simulink model "NREL_20MW_4TT"  (R2016b)
settings: Solver=VariableStepAuto  StartTime=0.0  StopTime=Tsim  FixedStep=Ts  RelTol=1e-3
values from neighbouring files (symbols this model uses):
  k1 = kgain(1,k)    [SimulationMain.m]
  k2 = kgain(2,k)    [SimulationMain.m]
  k3 = kgain(3,k)    [SimulationMain.m]
  k4 = kgain(4,k)    [SimulationMain.m]
  La02 = par.La02    [SimulationMain.m]
  La04 = par.La04    [SimulationMain.m]
  La12 = par.La12    [SimulationMain.m]
  La14 = par.La14    [SimulationMain.m]
  Lhub = par.Lhub    [SimulationMain.m]
  LNaz = par.LNaz    [SimulationMain.m]
  Lt1 = par.Lt1    [SimulationMain.m]
  Lt2 = par.Lt2    [SimulationMain.m]
  MRTCTs = 0.0125    [SimulationMain.m]
  NDOF = 10    [SimulationMain.m]
  Px_PowerMax = 6e6    (= 6000000)    [SimulationMain.m]
  ttilt = par.ttilt    [SimulationMain.m]
undefined symbols (not defined in the file or in its folder): A_4TT, B_4TT, C_4TT, D_4TT, DiscIntNeverNeededParam, Ks, Ms
libraries: libturbines x4
settings Model: Creator=kihso  LastModifiedBy=kihso

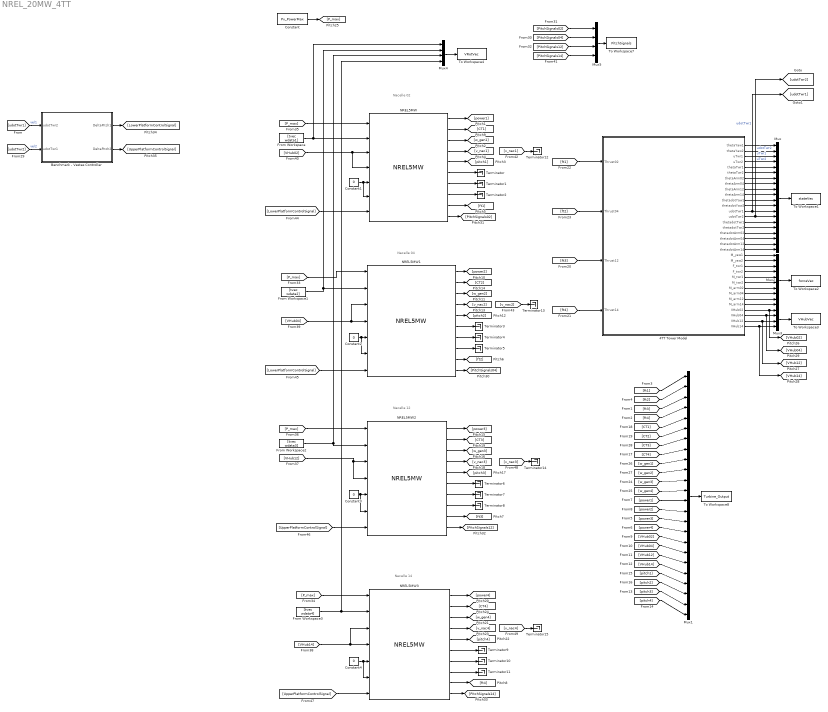
== system NREL_20MW_4TT ==
  note: Nacelle 02
  note: Nacelle 04
  note: Nacelle 12
  note: Nacelle 14
  4TT Tower Model : Subsystem
      mask Display: image(imread('Icon_4TT.JPG'))
  Benchmark - Vestas Controller : Subsystem
      mask Display: image('Vestas.png');
  Constant : Constant  Value=Px_PowerMax
  Constant{1..4} (4 alike) : Constant  Value=0
  From Workspace : FromWorkspace  VariableName=[tvec wdata1]  SampleTime=0
  From Workspace1 : FromWorkspace  VariableName=[tvec wdata2]  SampleTime=0
  From Workspace2 : FromWorkspace  VariableName=[tvec wdata3]  SampleTime=0
  From Workspace3 : FromWorkspace  VariableName=[tvec wdata4]  SampleTime=0
  Mux : Mux  Inputs=20
  Mux1 : Mux  Inputs=24
  Mux2 : Mux  Inputs=10
  Mux3 : Mux
  Mux4 : Mux
  Mux5 : Mux
  NREL5MW{,1..3} (4 alike) : libturbines/NREL5MW  w_gen_ini=50  beta_ini=0  auto=off  torq_ini=0
  Terminator{,1..15} (16 alike) : Terminator
  To Workspace1 : ToWorkspace  VariableName=stateVec  SaveFormat=Timeseries  SampleTime=-1
  To Workspace2 : ToWorkspace  VariableName=forceVec  SaveFormat=Timeseries  SampleTime=-1
  To Workspace3 : ToWorkspace  VariableName=VHubVec  SaveFormat=Timeseries  SampleTime=-1
  To Workspace4 : ToWorkspace  VariableName=VRotVec  SaveFormat=Timeseries  SampleTime=-1
  To Workspace7 : ToWorkspace  VariableName=PitchSignals  SaveFormat=Timeseries  SampleTime=-1
  To Workspace8 : ToWorkspace  VariableName=Turbine_Output  SaveFormat=Timeseries  SampleTime=-1
  wires:
  4TT Tower Model/1(thetaYaw1) -> Mux/1
  4TT Tower Model/2(thetaYaw2) -> Mux/2
  4TT Tower Model/3(uTwr1) -> Mux/3  "uTwr1"
  4TT Tower Model/4(uTwr2) -> Mux/4  "uTwr2"
  4TT Tower Model/5(thetaTwr1) -> Mux/5
  4TT Tower Model/6(thetaTwr2) -> Mux/6
  4TT Tower Model/7(thetaArm02) -> Mux/7
  4TT Tower Model/8(thetaArm04) -> Mux/8
  4TT Tower Model/9(thetaArm12) -> Mux/9
  4TT Tower Model/10(thetaArm14) -> Mux/10
  4TT Tower Model/11(thetadotYaw1) -> Mux/11
  4TT Tower Model/12(thetadotYaw2) -> Mux/12
  4TT Tower Model/13(udotTwr1) -> Mux/13, [udotTwr1]  "udotTwr1"
  4TT Tower Model/14(udotTwr2) -> Mux/14, [udotTwr2]  "udotTwr2"
  4TT Tower Model/15(thetadotTwr1) -> Mux/15
  4TT Tower Model/16(thetadotTwr2) -> Mux/16
  4TT Tower Model/17(thetadotArm02) -> Mux/17
  4TT Tower Model/18(thetadotArm04) -> Mux/18
  4TT Tower Model/19(thetadotArm12) -> Mux/19
  Mux1/1 -> To Workspace8/1
  NREL5MW/1 -> [power1]
  NREL5MW/2 -> [CT1]
  NREL5MW/3 -> [w_gen1]
  NREL5MW1/6 -> Terminator3/1
  NREL5MW1/7 -> Terminator4/1
  NREL5MW1/5 -> [pitch2]
  NREL5MW1/1 -> [power2]
  NREL5MW1/3 -> [w_gen2]
  NREL5MW1/2 -> [CT2]
  NREL5MW1/4 -> [v_nac2]
  NREL5MW2/6 -> Terminator6/1
  NREL5MW2/7 -> Terminator7/1
  NREL5MW2/2 -> [CT3]
  NREL5MW2/4 -> [v_nac3]
  NREL5MW2/1 -> [power3]
  NREL5MW2/3 -> [w_gen3]
  NREL5MW2/5 -> [pitch3]
  NREL5MW3/6 -> Terminator9/1
  NREL5MW3/7 -> Terminator10/1
  NREL5MW3/1 -> [power4]
  NREL5MW3/2 -> [CT4]
  NREL5MW3/4 -> [v_nac4]
  NREL5MW3/5 -> [pitch4]
  NREL5MW3/3 -> [w_gen4]
  Constant/1 -> [P_max]
  [P_max] -> NREL5MW/1
  [P_max] -> NREL5MW1/1
  [P_max] -> NREL5MW3/1
  From Workspace3/1 -> NREL5MW3/2, Mux4/4
  From Workspace2/1 -> NREL5MW2/2, Mux4/3
  From Workspace1/1 -> Mux4/2, NREL5MW1/2
  From Workspace/1 -> NREL5MW/2, Mux4/1
  4TT Tower Model/23(F_twr1) -> Mux2/3
  4TT Tower Model/26(M_twr2) -> Mux2/6
  4TT Tower Model/30(M_arm14) -> Mux2/10
  4TT Tower Model/28(M_arm04) -> Mux2/8
  4TT Tower Model/21(M_yaw1) -> Mux2/1
  4TT Tower Model/24(F_twr2) -> Mux2/4
  4TT Tower Model/25(M_twr1) -> Mux2/5
  4TT Tower Model/22(M_yaw2) -> Mux2/2
  4TT Tower Model/29(M_arm12) -> Mux2/9
  4TT Tower Model/27(M_arm02) -> Mux2/7
  Mux2/1 -> To Workspace2/1
  4TT Tower Model/31(VHub02) -> [VHub02], Mux3/1
  4TT Tower Model/32(VHub04) -> [VHub04], Mux3/2
  4TT Tower Model/33(VHub12) -> [VHub12], Mux3/3
  4TT Tower Model/34(VHub14) -> [VHub14], Mux3/4
  Mux3/1 -> To Workspace3/1
  [VHub04] -> NREL5MW1/4, NREL5MW1/3
  [VHub02] -> NREL5MW/4, NREL5MW/3
  NREL5MW1/8 -> Terminator5/1
  NREL5MW2/8 -> Terminator8/1
  NREL5MW3/8 -> Terminator11/1
  [ft1] -> 4TT Tower Model/1(Thrust02)
  [ft2] -> 4TT Tower Model/2(Thrust04)
  [ft3] -> 4TT Tower Model/3(Thrust12)
  [ft4] -> 4TT Tower Model/4(Thrust14)
  [VHub12] -> NREL5MW2/4, NREL5MW2/3
  [VHub14] -> NREL5MW3/4, NREL5MW3/3
  [w_gen1] -> Mux1/9
  [VHub04] -> Mux1/18
  [pitch2] -> Mux1/22
  [VHub12] -> Mux1/19
  [ft4] -> Mux1/4
  [ft1] -> Mux1/1
  [ft3] -> Mux1/3
  [CT4] -> Mux1/8
  [pitch3] -> Mux1/23
  [pitch4] -> Mux1/24
  [CT1] -> Mux1/5
  [CT2] -> Mux1/6
  [w_gen4] -> Mux1/12
  [pitch1] -> Mux1/21
  [w_gen2] -> Mux1/10
  [CT3] -> Mux1/7
  [VHub02] -> Mux1/17
  [w_gen3] -> Mux1/11
  [VHub14] -> Mux1/20
  [ft2] -> Mux1/2
  [power3] -> Mux1/15
  [power1] -> Mux1/13
  [power4] -> Mux1/16
  [power2] -> Mux1/14
  NREL5MW1/9 -> [ft2]
  NREL5MW2/9 -> [ft3]
  NREL5MW3/9 -> [ft4]
  Mux/1 -> To Workspace1/1
  Mux4/1 -> To Workspace4/1
  NREL5MW/4 -> [v_nac1]
  NREL5MW/5 -> [pitch1]
  NREL5MW/6 -> Terminator/1
  NREL5MW/7 -> Terminator1/1
  NREL5MW/8 -> Terminator2/1
  NREL5MW/9 -> [ft1]
  [P_max] -> NREL5MW2/1
  NREL5MW2/10 -> [PitchSignals12]
  NREL5MW3/10 -> [PitchSignals14]
  [PitchSignals02] -> Mux5/1
  [PitchSignals14] -> Mux5/4
  [PitchSignals04] -> Mux5/2
  [PitchSignals12] -> Mux5/3
  Mux5/1 -> To Workspace7/1
  4TT Tower Model/20(thetadotArm14) -> Mux/20
  [LowerPlatformControlSignal] -> NREL5MW/7
  [LowerPlatformControlSignal] -> NREL5MW1/7
  [UpperPlatformControlSignal] -> NREL5MW2/7
  [UpperPlatformControlSignal] -> NREL5MW3/7
  NREL5MW/10 -> [PitchSignals02]
  NREL5MW1/10 -> [PitchSignals04]
  Benchmark - Vestas Controller/1(DeltaPitch1) -> [LowerPlatformControlSignal]
  Benchmark - Vestas Controller/2(DeltaPitch2) -> [UpperPlatformControlSignal]
  [udotTwr1] -> Benchmark - Vestas Controller/1(udotTwr2)  "vel1"
  [udotTwr2] -> Benchmark - Vestas Controller/2(udotTwr1)  "vel2"
  Constant1/1 -> NREL5MW/6, NREL5MW/5
  Constant2/1 -> NREL5MW1/6, NREL5MW1/5
  Constant3/1 -> NREL5MW2/6, NREL5MW2/5
  Constant4/1 -> NREL5MW3/6, NREL5MW3/5
  [v_nac1] -> Terminator12/1
  [v_nac2] -> Terminator13/1
  [v_nac3] -> Terminator14/1
  [v_nac4] -> Terminator15/1

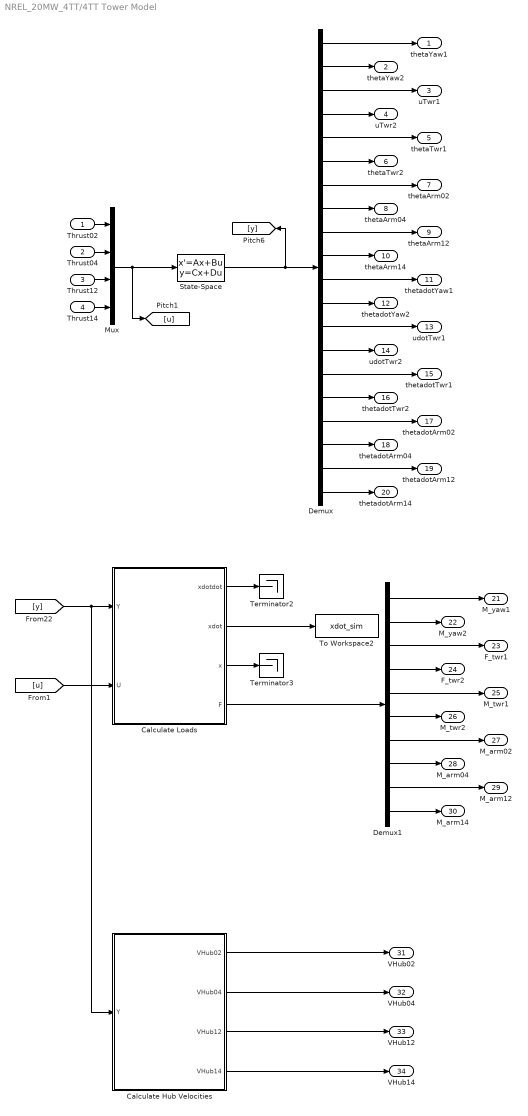
== system NREL_20MW_4TT/4TT Tower Model ==
  Thrust02 : Inport
  Thrust04 : Inport  Port=2
  Thrust12 : Inport  Port=3
  Thrust14 : Inport  Port=4
  Calculate Hub Velocities : MATLAB Function  TreatAsAtomicUnit=on
  Calculate Loads : MATLAB Function  TreatAsAtomicUnit=on
  Demux : Demux  Outputs=20
  Demux1 : Demux  Outputs=10
  Mux : Mux
  State-Space : StateSpace  A=A_4TT  B=B_4TT  C=C_4TT  D=D_4TT  X0=0
  Terminator2 : Terminator
  Terminator3 : Terminator
  To Workspace2 : ToWorkspace  VariableName=xdot_sim  SaveFormat=Timeseries  SampleTime=-1
  thetaYaw1 : Outport
  thetaYaw2 : Outport  Port=2
  uTwr1 : Outport  Port=3
  uTwr2 : Outport  Port=4
  thetaTwr1 : Outport  Port=5
  thetaTwr2 : Outport  Port=6
  thetaArm02 : Outport  Port=7
  thetaArm04 : Outport  Port=8
  thetaArm12 : Outport  Port=9
  thetaArm14 : Outport  Port=10
  thetadotYaw1 : Outport  Port=11
  thetadotYaw2 : Outport  Port=12
  udotTwr1 : Outport  Port=13
  udotTwr2 : Outport  Port=14
  thetadotTwr1 : Outport  Port=15
  thetadotTwr2 : Outport  Port=16
  thetadotArm02 : Outport  Port=17
  thetadotArm04 : Outport  Port=18
  thetadotArm12 : Outport  Port=19
  thetadotArm14 : Outport  Port=20
  M_yaw1 : Outport  Port=21
  M_yaw2 : Outport  Port=22
  F_twr1 : Outport  Port=23
  F_twr2 : Outport  Port=24
  M_twr1 : Outport  Port=25
  M_twr2 : Outport  Port=26
  M_arm02 : Outport  Port=27
  M_arm04 : Outport  Port=28
  M_arm12 : Outport  Port=29
  M_arm14 : Outport  Port=30
  VHub02 : Outport  Port=31
  VHub04 : Outport  Port=32
  VHub12 : Outport  Port=33
  VHub14 : Outport  Port=34
  wires:
  State-Space/1 -> [y], Demux/1
  Mux/1 -> [u], State-Space/1
  Demux/6 -> thetaTwr2/1
  Demux/7 -> thetaArm02/1
  Thrust14/1 -> Mux/4
  Demux/4 -> uTwr2/1
  Demux/2 -> thetaYaw2/1
  Demux/1 -> thetaYaw1/1
  Demux/3 -> uTwr1/1
  Demux/8 -> thetaArm04/1
  Thrust12/1 -> Mux/3
  Demux/5 -> thetaTwr1/1
  Thrust04/1 -> Mux/2
  Thrust02/1 -> Mux/1
  Demux/9 -> thetaArm12/1
  Demux/10 -> thetaArm14/1
  Demux/16 -> thetadotTwr2/1
  Demux/17 -> thetadotArm02/1
  Demux/14 -> udotTwr2/1
  Demux/12 -> thetadotYaw2/1
  Demux/11 -> thetadotYaw1/1
  Demux/13 -> udotTwr1/1
  Demux/18 -> thetadotArm04/1
  Demux/15 -> thetadotTwr1/1
  Demux/19 -> thetadotArm12/1
  Demux/20 -> thetadotArm14/1
  [y] -> Calculate Hub Velocities/1(Y), Calculate Loads/1(Y)
  [u] -> Calculate Loads/2(U)
  Calculate Loads/4(F) -> Demux1/1
  Demux1/7 -> M_arm02/1
  Demux1/1 -> M_yaw1/1
  Demux1/3 -> F_twr1/1
  Demux1/5 -> M_twr1/1
  Demux1/9 -> M_arm12/1
  Demux1/2 -> M_yaw2/1
  Demux1/4 -> F_twr2/1
  Demux1/6 -> M_twr2/1
  Demux1/8 -> M_arm04/1
  Demux1/10 -> M_arm14/1
  Calculate Hub Velocities/4(VHub14) -> VHub14/1
  Calculate Hub Velocities/2(VHub04) -> VHub04/1
  Calculate Hub Velocities/3(VHub12) -> VHub12/1
  Calculate Hub Velocities/1(VHub02) -> VHub02/1
  Calculate Loads/2(xdot) -> To Workspace2/1
  Calculate Loads/1(xdotdot) -> Terminator2/1
  Calculate Loads/3(x) -> Terminator3/1

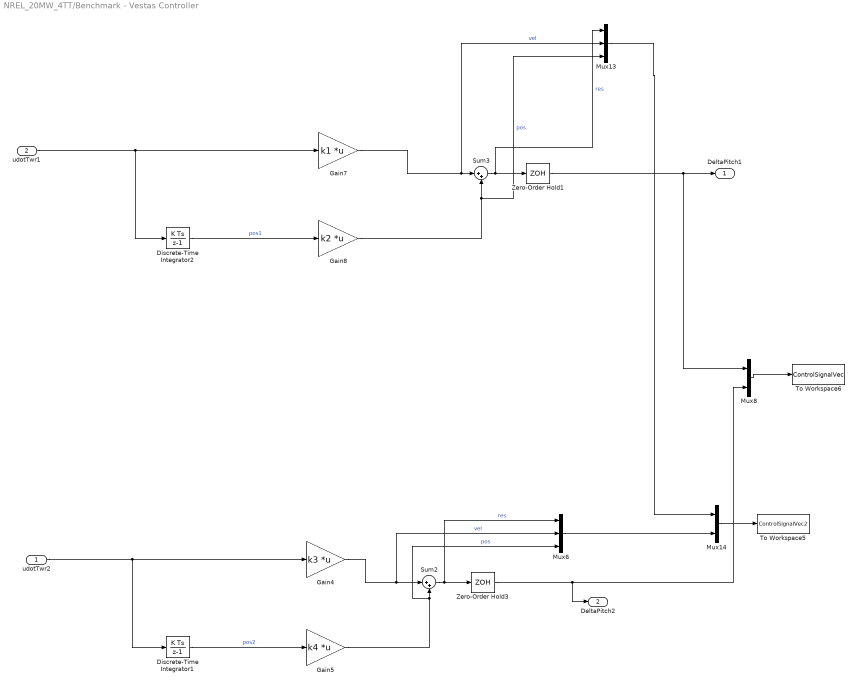
== system NREL_20MW_4TT/Benchmark - Vestas Controller ==
  udotTwr2 : Inport
  udotTwr1 : Inport  Port=2
  Discrete-Time Integrator1 : DiscreteIntegrator  InitialConditionSetting=State (most efficient)  SampleTime=-1  ICPrevOutput=DiscIntNeverNeededParam  ICPrevScaledInput=DiscIntNeverNeededParam
  Discrete-Time Integrator2 : DiscreteIntegrator  InitialConditionSetting=State (most efficient)  SampleTime=-1  ICPrevOutput=DiscIntNeverNeededParam  ICPrevScaledInput=DiscIntNeverNeededParam
  Gain4 : Gain  Gain=k3  Multiplication=Matrix(K*u)
  Gain5 : Gain  Gain=k4  Multiplication=Matrix(K*u)
  Gain7 : Gain  Gain=k1  Multiplication=Matrix(K*u)
  Gain8 : Gain  Gain=k2  Multiplication=Matrix(K*u)
  Mux13 : Mux  Inputs=3
  Mux14 : Mux  Inputs=2
  Mux6 : Mux  Inputs=3
  Mux8 : Mux  Inputs=2
  Sum2 : Sum  Inputs=|++
  Sum3 : Sum  Inputs=|++
  To Workspace5 : ToWorkspace  VariableName=ControlSignalVec2  SaveFormat=Timeseries  SampleTime=-1
  To Workspace6 : ToWorkspace  VariableName=ControlSignalVec  SaveFormat=Timeseries  SampleTime=-1
  Zero-Order Hold1 : ZeroOrderHold  SampleTime=MRTCTs
  Zero-Order Hold3 : ZeroOrderHold  SampleTime=MRTCTs
  DeltaPitch1 : Outport
  DeltaPitch2 : Outport  Port=2
  wires:
  Mux13/1 -> Mux14/1
  Sum2/1 -> Zero-Order Hold3/1, Mux6/1  "res"
  Sum3/1 -> Mux13/1, Zero-Order Hold1/1  "res"
  Discrete-Time Integrator2/1 -> Gain8/1  "pos1"
  udotTwr2/1 -> Discrete-Time Integrator1/1, Gain4/1
  Gain5/1 -> Mux6/3, Sum2/2  "pos"
  Mux8/1 -> To Workspace6/1
  Mux6/1 -> Mux14/2
  udotTwr1/1 -> Discrete-Time Integrator2/1, Gain7/1
  Gain8/1 -> Mux13/3, Sum3/2  "pos"
  Discrete-Time Integrator1/1 -> Gain5/1  "pos2"
  Gain7/1 -> Mux13/2, Sum3/1  "vel"
  Gain4/1 -> Sum2/1, Mux6/2  "vel"
  Mux14/1 -> To Workspace5/1
  Zero-Order Hold1/1 -> Mux8/1, DeltaPitch1/1
  Zero-Order Hold3/1 -> Mux8/2, DeltaPitch2/1

== matlab function NREL_20MW_4TT/4TT Tower Model/Calculate Hub Velocities ==
  data: VHub02 output; VHub04 output; Y input; VHub12 output; VHub14 output; ttilt parameter; La02 parameter; La04 parameter; La12 parameter; La14 parameter; Lhub parameter; LNaz parameter; NDOF parameter; Lt1 parameter; Lt2 parameter
 function [VHub02,VHub04,VHub12,VHub14]=HubVel(Y,ttilt, La02, La04, La12, La14, Lhub, LNaz, NDOF,Lt1, Lt2)

x=Y(1:NDOF,1);
xdot=Y(NDOF+1:NDOF*2,1);

% Position dofs
x1=x(1);
x2=x(2);
x3=x(3);
x4=x(4);
x5=x(5);
x6=x(6);
x7=x(7);
x8=x(8);
x9=x(9);
x10=x(10);
% Velocity dofs
dxdt1=xdot(1);
dxdt2=xdot(2);
dxdt3=xdot(3);
dxdt4=xdot(4);
dxdt5=xdot(5);
dxdt6=xdot(6);
dxdt7=xdot(7);
dxdt8=xdot(8);
dxdt9=xdot(9);
dxdt10=xdot(10);

% % Geometrical properties
% ttilt=par.ttilt;
% La02=par.La02;
% La04=par.La04;
% La12=par.La12;
% La14=par.La14;
% Lhub=par.Lhub;
% LNaz=par.LNaz;

% Hub velocities
VHub02_xyz =[dxdt1 * sin(x1) * La02 + dxdt1 * cos(x1) * (-cos(x7) * cos(ttilt) * Lhub + sin(x7) * (-LNaz + sin(ttilt) * Lhub)) + sin(x1) * (dxdt7 * sin(x7) * cos(ttilt) * Lhub + dxdt7 * cos(x7) * (-LNaz + sin(ttilt) * Lhub)) dxdt3 - dxdt5 * sin(x5) * sin(x1) * La02 + cos(x5) * dxdt1 * cos(x1) * La02 - dxdt5 * sin(x5) * cos(x1) * (-cos(x7) * cos(ttilt) * Lhub + sin(x7) * (-LNaz + sin(ttilt) * Lhub)) - cos(x5) * dxdt1 * sin(x1) * (-cos(x7) * cos(ttilt) * Lhub + sin(x7) * (-LNaz + sin(ttilt) * Lhub)) + cos(x5) * cos(x1) * (dxdt7 * sin(x7) * cos(ttilt) * Lhub + dxdt7 * cos(x7) * (-LNaz + sin(ttilt) * Lhub)) + dxdt5 * cos(x5) * (sin(x7) * cos(ttilt) * Lhub + cos(x7) * (-LNaz + sin(ttilt) * Lhub)) + sin(x5) * (dxdt7 * cos(x7) * cos(ttilt) * Lhub - dxdt7 * sin(x7) * (-LNaz + sin(ttilt) * Lhub)) -dxdt5 * cos(x5) * sin(x1) * La02 - sin(x5) * dxdt1 * cos(x1) * La02 - dxdt5 * cos(x5) * cos(x1) * (-cos(x7) * cos(ttilt) * Lhub + sin(x7) * (-LNaz + sin(ttilt) * Lhub)) + sin(x5) * dxdt1 * sin(x1) * (-cos(x7) * cos(ttilt) * Lhub + sin(x7) * (-LNaz + sin(ttilt) * Lhub)) - sin(x5) * cos(x1) * (dxdt7 * sin(x7) * cos(ttilt) * Lhub + dxdt7 * cos(x7) * (-LNaz + sin(ttilt) * Lhub)) - dxdt5 * sin(x5) * (sin(x7) * cos(ttilt) * Lhub + cos(x7) * (-LNaz + sin(ttilt) * Lhub)) + cos(x5) * (dxdt7 * cos(x7) * cos(ttilt) * Lhub - dxdt7 * sin(x7) * (-LNaz + sin(ttilt) * Lhub))];

VHub04_xyz = [-dxdt1 * sin(x1) * La04 + dxdt1 * cos(x1) * (-cos(x8) * cos(ttilt) * Lhub + sin(x8) * (-LNaz + sin(ttilt) * Lhub)) + sin(x1) * (dxdt8 * sin(x8) * cos(ttilt) * Lhub + dxdt8 * cos(x8) * (-LNaz + sin(ttilt) * Lhub)) dxdt3 + dxdt5 * sin(x5) * sin(x1) * La04 - cos(x5) * dxdt1 * cos(x1) * La04 - dxdt5 * sin(x5) * cos(x1) * (-cos(x8) * cos(ttilt) * Lhub + sin(x8) * (-LNaz + sin(ttilt) * Lhub)) - cos(x5) * dxdt1 * sin(x1) * (-cos(x8) * cos(ttilt) * Lhub + sin(x8) * (-LNaz + sin(ttilt) * Lhub)) + cos(x5) * cos(x1) * (dxdt8 * sin(x8) * cos(ttilt) * Lhub + dxdt8 * cos(x8) * (-LNaz + sin(ttilt) * Lhub)) + dxdt5 * cos(x5) * (sin(x8) * cos(ttilt) * Lhub + cos(x8) * (-LNaz + sin(ttilt) * Lhub)) + sin(x5) * (dxdt8 * cos(x8) * cos(ttilt) * Lhub - dxdt8 * sin(x8) * (-LNaz + sin(ttilt) * Lhub)) dxdt5 * cos(x5) * sin(x1) * La04 + sin(x5) * dxdt1 * cos(x1) * La04 - dxdt5 * cos(x5) * cos(x1) * (-cos(x8) * cos(ttilt) * Lhub + sin(x8) * (-LNaz + sin(ttilt) * Lhub)) + sin(x5) * dxdt1 * sin(x1) * (-cos(x8) * cos(ttilt) * Lhub + sin(x8) * (-LNaz + sin(ttilt) * Lhub)) - sin(x5) * cos(x1) * (dxdt8 * sin(x8) * cos(ttilt) * Lhub + dxdt8 * cos(x8) * (-LNaz + sin(ttilt) * Lhub)) - dxdt5 * sin(x5) * (sin(x8) * cos(ttilt) * Lhub + cos(x8) * (-LNaz + sin(ttilt) * Lhub)) + cos(x5) * (dxdt8 * cos(x8) * cos(ttilt) * Lhub - dxdt8 * sin(x8) * (-LNaz + sin(ttilt) * Lhub))];

VHub12_xyz = [-dxdt1 * sin(x1) * (-cos(x2) * La12 + sin(x2) * (-cos(x9) * cos(ttilt) * Lhub + sin(x9) * (-LNaz + sin(ttilt) * Lhub))) + cos(x1) * (dxdt2 * sin(x2) * La12 + dxdt2 * cos(x2) * (-cos(x9) * cos(ttilt) * Lhub + sin(x9) * (-LNaz + sin(ttilt) * Lhub)) + sin(x2) * (dxdt9 * sin(x9) * cos(ttilt) * Lhub + dxdt9 * cos(x9) * (-LNaz + sin(ttilt) * Lhub))) + dxdt1 * cos(x1) * (x4 + cos(x6) * sin(x2) * La12 + cos(x6) * cos(x2) * (-cos(x9) * cos(ttilt) * Lhub + sin(x9) * (-LNaz + sin(ttilt) * Lhub)) + sin(x6) * (sin(x9) * cos(ttilt) * Lhub + cos(x9) * (-LNaz + sin(ttilt) * Lhub))) + sin(x1) * (dxdt4 - dxdt6 * sin(x6) * sin(x2) * La12 + cos(x6) * dxdt2 * cos(x2) * La12 - dxdt6 * sin(x6) * cos(x2) * (-cos(x9) * cos(ttilt) * Lhub + sin(x9) * (-LNaz + sin(ttilt) * Lhub)) - cos(x6) * dxdt2 * sin(x2) * (-cos(x9) * cos(ttilt) * Lhub + sin(x9) * (-LNaz + sin(ttilt) * Lhub)) + cos(x6) * cos(x2) * (dxdt9 * sin(x9) * cos(ttilt) * Lhub + dxdt9 * cos(x9) * (-LNaz + sin(ttilt) * Lhub)) + dxdt6 * cos(x6) * (sin(x9) * cos(ttilt) * Lhub + cos(x9) * (-LNaz + sin(ttilt) * Lhub)) + sin(x6) * (dxdt9 * cos(x9) * cos(ttilt) * Lhub - dxdt9 * sin(x9) * (-LNaz + sin(ttilt) * Lhub))) dxdt3 + dxdt5 * sin(x5) * sin(x1) * (-cos(x2) * La12 + sin(x2) * (-cos(x9) * cos(ttilt) * Lhub + sin(x9) * (-LNaz + sin(ttilt) * Lhub))) - cos(x5) * dxdt1 * cos(x1) * (-cos(x2) * La12 + sin(x2) * (-cos(x9) * cos(ttilt) * Lhub + sin(x9) * (-LNaz + sin(ttilt) * Lhub))) - cos(x5) * sin(x1) * (dxdt2 * sin(x2) * La12 + dxdt2 * cos(x2) * (-cos(x9) * cos(ttilt) * Lhub + sin(x9) * (-LNaz + sin(ttilt) * Lhub)) + sin(x2) * (dxdt9 * sin(x9) * cos(ttilt) * Lhub + dxdt9 * cos(x9) * (-LNaz + sin(ttilt) * Lhub))) - dxdt5 * sin(x5) * cos(x1) * (x4 + cos(x6) * sin(x2) * La12 + cos(x6) * cos(x2) * (-cos(x9) * cos(ttilt) * Lhub + sin(x9) * (-LNaz + sin(ttilt) * Lhub)) + sin(x6) * (sin(x9) * cos(ttilt) * Lhub + cos(x9) * (-LNaz + sin(ttilt) * Lhub))) - cos(x5) * dxdt1 * sin(x1) * (x4 + cos(x6) * sin(x2) * La12 + cos(x6) * cos(x2) * (-cos(x9) * cos(ttilt) * Lhub + sin(x9) * (-LNaz + sin(ttilt) * Lhub)) + sin(x6) * (sin(x9) * cos(ttilt) * Lhub + cos(x9) * (-LNaz + sin(ttilt) * Lhub))) + cos(x5) * cos(x1) * (dxdt4 - dxdt6 * sin(x6) * sin(x2) * La12 + cos(x6) * dxdt2 * cos(x2) * La12 - dxdt6 * sin(x6) * cos(x2) * (-cos(x9) * cos(ttilt) * Lhub + sin(x9) * (-LNaz + sin(ttilt) * Lhub)) - cos(x6) * dxdt2 * sin(x2) * (-cos(x9) * cos(ttilt) * Lhub + sin(x9) * (-LNaz + sin(ttilt) * Lhub)) + cos(x6) * cos(x2) * (dxdt9 * sin(x9) * cos(ttilt) * Lhub + dxdt9 * cos(x9) * (-LNaz + sin(ttilt) * Lhub)) + dxdt6 * cos(x6) * (sin(x9) * cos(ttilt) * Lhub + cos(x9) * (-LNaz + sin(ttilt) * Lhub)) + sin(x6) * (dxdt9 * cos(x9) * cos(ttilt) * Lhub - dxdt9 * sin(x9) * (-LNaz + sin(ttilt) * Lhub))) + dxdt5 * cos(x5) * (-Lt2 - sin(x6) * sin(x2) * La12 - sin(x6) * cos(x2) * (-cos(x9) * cos(ttilt) * Lhub + sin(x9) * (-LNaz + sin(ttilt) * Lhub)) + cos(x6) * (sin(x9) * cos(ttilt) * Lhub + cos(x9) * (-LNaz + sin(ttilt) * Lhub))) + sin(x5) * (-dxdt6 * cos(x6) * sin(x2) * La12 - sin(x6) * dxdt2 * cos(x2) * La12 - dxdt6 * cos(x6) * cos(x2) * (-cos(x9) * cos(ttilt) * Lhub + sin(x9) * (-LNaz + sin(ttilt) * Lhub)) + sin(x6) * dxdt2 * sin(x2) * (-cos(x9) * cos(ttilt) * Lhub + sin(x9) * (-LNaz + sin(ttilt) * Lhub)) - sin(x6) * cos(x2) * (dxdt9 * sin(x9) * cos(ttilt) * Lhub + dxdt9 * cos(x9) * (-LNaz + sin(ttilt) * Lhub)) - dxdt6 * sin(x6) * (sin(x9) * cos(ttilt) * Lhub + cos(x9) * (-LNaz + sin(ttilt) * Lhub)) + cos(x6) * (dxdt9 * cos(x9) * cos(ttilt) * Lhub - dxdt9 * sin(x9) * (-LNaz + sin(ttilt) * Lhub))) dxdt5 * cos(x5) * sin(x1) * (-cos(x2) * La12 + sin(x2) * (-cos(x9) * cos(ttilt) * Lhub + sin(x9) * (-LNaz + sin(ttilt) * Lhub))) + sin(x5) * dxdt1 * cos(x1) * (-cos(x2) * La12 + sin(x2) * (-cos(x9) * cos(ttilt) * Lhub + sin(x9) * (-LNaz + sin(ttilt) * Lhub))) + sin(x5) * sin(x1) * (dxdt2 * sin(x2) * La12 + dxdt2 * cos(x2) * (-cos(x9) * cos(ttilt) * Lhub + sin(x9) * (-LNaz + sin(ttilt) * Lhub)) + sin(x2) * (dxdt9 * sin(x9) * cos(ttilt) * Lhub + dxdt9 * cos(x9) * (-LNaz + sin(ttilt) * Lhub))) - dxdt5 * cos(x5) * cos(x1) * (x4 + cos(x6) * sin(x2) * La12 + cos(x6) * cos(x2) * (-cos(x9) * cos(ttilt) * Lhub + sin(x9) * (-LNaz + sin(ttilt) * Lhub)) + sin(x6) * (sin(x9) * cos(ttilt) * Lhub + cos(x9) * (-LNaz + sin(ttilt) * Lhub))) + sin(x5) * dxdt1 * sin(x1) * (x4 + cos(x6) * sin(x2) * La12 + cos(x6) * cos(x2) * (-cos(x9) * cos(ttilt) * Lhub + sin(x9) * (-LNaz + sin(ttilt) * Lhub)) + sin(x6) * (sin(x9) * cos(ttilt) * Lhub + cos(x9) * (-LNaz + sin(ttilt) * Lhub))) - sin(x5) * cos(x1) * (dxdt4 - dxdt6 * sin(x6) * sin(x2) * La12 + cos(x6) * dxdt2 * cos(x2) * La12 - dxdt6 * sin(x6) * cos(x2) * (-cos(x9) * cos(ttilt) * Lhub + sin(x9) * (-LNaz + sin(ttilt) * Lhub)) - cos(x6) * dxdt2 * sin(x2) * (-cos(x9) * cos(ttilt) * Lhub + sin(x9) * (-LNaz + sin(ttilt) * Lhub)) + cos(x6) * cos(x2) * (dxdt9 * sin(x9) * cos(ttilt) * Lhub + dxdt9 * cos(x9) * (-LNaz + sin(ttilt) * Lhub)) + dxdt6 * cos(x6) * (sin(x9) * cos(ttilt) * Lhub + cos(x9) * (-LNaz + sin(ttilt) * Lhub)) + sin(x6) * (dxdt9 * cos(x9) * cos(ttilt) * Lhub - dxdt9 * sin(x9) * (-LNaz + sin(ttilt) * Lhub))) - dxdt5 * sin(x5) * (-Lt2 - sin(x6) * sin(x2) * La12 - sin(x6) * cos(x2) * (-cos(x9) * cos(ttilt) * Lhub + sin(x9) * (-LNaz + sin(ttilt) * Lhub)) + cos(x6) * (sin(x9) * cos(ttilt) * Lhub + cos(x9) * (-LNaz + sin(ttilt) * Lhub))) + cos(x5) * (-dxdt6 * cos(x6) * sin(x2) * La12 - sin(x6) * dxdt2 * cos(x2) * La12 - dxdt6 * cos(x6) * cos(x2) * (-cos(x9) * cos(ttilt) * Lhub + sin(x9) * (-LNaz + sin(ttilt) * Lhub)) + sin(x6) * dxdt2 * sin(x2) * (-cos(x9) * cos(ttilt) * Lhub + sin(x9) * (-LNaz + sin(ttilt) * Lhub)) - sin(x6) * cos(x2) * (dxdt9 * sin(x9) * cos(ttilt) * Lhub + dxdt9 * cos(x9) * (-LNaz + sin(ttilt) * Lhub)) - dxdt6 * sin(x6) * (sin(x9) * cos(ttilt) * Lhub + cos(x9) * (-LNaz + sin(ttilt) * Lhub)) + cos(x6) * (dxdt9 * cos(x9) * cos(ttilt) * Lhub - dxdt9 * sin(x9) * (-LNaz + sin(ttilt) * Lhub)))];

VHub14_xyz = [-dxdt1 * sin(x1) * (cos(x2) * La14 + sin(x2) * (-cos(x10) * cos(ttilt) * Lhub + sin(x10) * (-LNaz + sin(ttilt) * Lhub))) + cos(x1) * (-dxdt2 * sin(x2) * La14 + dxdt2 * cos(x2) * (-cos(x10) * cos(ttilt) * Lhub + sin(x10) * (-LNaz + sin(ttilt) * Lhub)) + sin(x2) * (dxdt10 * sin(x10) * cos(ttilt) * Lhub + dxdt10 * cos(x10) * (-LNaz + sin(ttilt) * Lhub))) + dxdt1 * cos(x1) * (x4 - cos(x6) * sin(x2) * La14 + cos(x6) * cos(x2) * (-cos(x10) * cos(ttilt) * Lhub + sin(x10) * (-LNaz + sin(ttilt) * Lhub)) + sin(x6) * (sin(x10) * cos(ttilt) * Lhub + cos(x10) * (-LNaz + sin(ttilt) * Lhub))) + sin(x1) * (dxdt4 + dxdt6 * sin(x6) * sin(x2) * La14 - cos(x6) * dxdt2 * cos(x2) * La14 - dxdt6 * sin(x6) * cos(x2) * (-cos(x10) * cos(ttilt) * Lhub + sin(x10) * (-LNaz + sin(ttilt) * Lhub)) - cos(x6) * dxdt2 * sin(x2) * (-cos(x10) * cos(ttilt) * Lhub + sin(x10) * (-LNaz + sin(ttilt) * Lhub)) + cos(x6) * cos(x2) * (dxdt10 * sin(x10) * cos(ttilt) * Lhub + dxdt10 * cos(x10) * (-LNaz + sin(ttilt) * Lhub)) + dxdt6 * cos(x6) * (sin(x10) * cos(ttilt) * Lhub + cos(x10) * (-LNaz + sin(ttilt) * Lhub)) + sin(x6) * (dxdt10 * cos(x10) * cos(ttilt) * Lhub - dxdt10 * sin(x10) * (-LNaz + sin(ttilt) * Lhub))) dxdt3 + dxdt5 * sin(x5) * sin(x1) * (cos(x2) * La14 + sin(x2) * (-cos(x10) * cos(ttilt) * Lhub + sin(x10) * (-LNaz + sin(ttilt) * Lhub))) - cos(x5) * dxdt1 * cos(x1) * (cos(x2) * La14 + sin(x2) * (-cos(x10) * cos(ttilt) * Lhub + sin(x10) * (-LNaz + sin(ttilt) * Lhub))) - cos(x5) * sin(x1) * (-dxdt2 * sin(x2) * La14 + dxdt2 * cos(x2) * (-cos(x10) * cos(ttilt) * Lhub + sin(x10) * (-LNaz + sin(ttilt) * Lhub)) + sin(x2) * (dxdt10 * sin(x10) * cos(ttilt) * Lhub + dxdt10 * cos(x10) * (-LNaz + sin(ttilt) * Lhub))) - dxdt5 * sin(x5) * cos(x1) * (x4 - cos(x6) * sin(x2) * La14 + cos(x6) * cos(x2) * (-cos(x10) * cos(ttilt) * Lhub + sin(x10) * (-LNaz + sin(ttilt) * Lhub)) + sin(x6) * (sin(x10) * cos(ttilt) * Lhub + cos(x10) * (-LNaz + sin(ttilt) * Lhub))) - cos(x5) * dxdt1 * sin(x1) * (x4 - cos(x6) * sin(x2) * La14 + cos(x6) * cos(x2) * (-cos(x10) * cos(ttilt) * Lhub + sin(x10) * (-LNaz + sin(ttilt) * Lhub)) + sin(x6) * (sin(x10) * cos(ttilt) * Lhub + cos(x10) * (-LNaz + sin(ttilt) * Lhub))) + cos(x5) * cos(x1) * (dxdt4 + dxdt6 * sin(x6) * sin(x2) * La14 - cos(x6) * dxdt2 * cos(x2) * La14 - dxdt6 * sin(x6) * cos(x2) * (-cos(x10) * cos(ttilt) * Lhub + sin(x10) * (-LNaz + sin(ttilt) * Lhub)) - cos(x6) * dxdt2 * sin(x2) * (-cos(x10) * cos(ttilt) * Lhub + sin(x10) * (-LNaz + sin(ttilt) * Lhub)) + cos(x6) * cos(x2) * (dxdt10 * sin(x10) * cos(ttilt) * Lhub + dxdt10 * cos(x10) * (-LNaz + sin(ttilt) * Lhub)) + dxdt6 * cos(x6) * (sin(x10) * cos(ttilt) * Lhub + cos(x10) * (-LNaz + sin(ttilt) * Lhub)) + sin(x6) * (dxdt10 * cos(x10) * cos(ttilt) * Lhub - dxdt10 * sin(x10) * (-LNaz + sin(ttilt) * Lhub))) + dxdt5 * cos(x5) * (-Lt2 + sin(x6) * sin(x2) * La14 - sin(x6) * cos(x2) * (-cos(x10) * cos(ttilt) * Lhub + sin(x10) * (-LNaz + sin(ttilt) * Lhub)) + cos(x6) * (sin(x10) * cos(ttilt) * Lhub + cos(x10) * (-LNaz + sin(ttilt) * Lhub))) + sin(x5) * (dxdt6 * cos(x6) * sin(x2) * La14 + sin(x6) * dxdt2 * cos(x2) * La14 - dxdt6 * cos(x6) * cos(x2) * (-cos(x10) * cos(ttilt) * Lhub + sin(x10) * (-LNaz + sin(ttilt) * Lhub)) + sin(x6) * dxdt2 * sin(x2) * (-cos(x10) * cos(ttilt) * Lhub + sin(x10) * (-LNaz + sin(ttilt) * Lhub)) - sin(x6) * cos(x2) * (dxdt10 * sin(x10) * cos(ttilt) * Lhub + dxdt10 * cos(x10) * (-LNaz + sin(ttilt) * Lhub)) - dxdt6 * sin(x6) * (sin(x10) * cos(ttilt) * Lhub + cos(x10) * (-LNaz + sin(ttilt) * Lhub)) + cos(x6) * (dxdt10 * cos(x10) * cos(ttilt) * Lhub - dxdt10 * sin(x10) * (-LNaz + sin(ttilt) * Lhub))) dxdt5 * cos(x5) * sin(x1) * (cos(x2) * La14 + sin(x2) * (-cos(x10) * cos(ttilt) * Lhub + sin(x10) * (-LNaz + sin(ttilt) * Lhub))) + sin(x5) * dxdt1 * cos(x1) * (cos(x2) * La14 + sin(x2) * (-cos(x10) * cos(ttilt) * Lhub + sin(x10) * (-LNaz + sin(ttilt) * Lhub))) + sin(x5) * sin(x1) * (-dxdt2 * sin(x2) * La14 + dxdt2 * cos(x2) * (-cos(x10) * cos(ttilt) * Lhub + sin(x10) * (-LNaz + sin(ttilt) * Lhub)) + sin(x2) * (dxdt10 * sin(x10) * cos(ttilt) * Lhub + dxdt10 * cos(x10) * (-LNaz + sin(ttilt) * Lhub))) - dxdt5 * cos(x5) * cos(x1) * (x4 - cos(x6) * sin(x2) * La14 + cos(x6) * cos(x2) * (-cos(x10) * cos(ttilt) * Lhub + sin(x10) * (-LNaz + sin(ttilt) * Lhub)) + sin(x6) * (sin(x10) * cos(ttilt) * Lhub + cos(x10) * (-LNaz + sin(ttilt) * Lhub))) + sin(x5) * dxdt1 * sin(x1) * (x4 - cos(x6) * sin(x2) * La14 + cos(x6) * cos(x2) * (-cos(x10) * cos(ttilt) * Lhub + sin(x10) * (-LNaz + sin(ttilt) * Lhub)) + sin(x6) * (sin(x10) * cos(ttilt) * Lhub + cos(x10) * (-LNaz + sin(ttilt) * Lhub))) - sin(x5) * cos(x1) * (dxdt4 + dxdt6 * sin(x6) * sin(x2) * La14 - cos(x6) * dxdt2 * cos(x2) * La14 - dxdt6 * sin(x6) * cos(x2) * (-cos(x10) * cos(ttilt) * Lhub + sin(x10) * (-LNaz + sin(ttilt) * Lhub)) - cos(x6) * dxdt2 * sin(x2) * (-cos(x10) * cos(ttilt) * Lhub + sin(x10) * (-LNaz + sin(ttilt) * Lhub)) + cos(x6) * cos(x2) * (dxdt10 * sin(x10) * cos(ttilt) * Lhub + dxdt10 * cos(x10) * (-LNaz + sin(ttilt) * Lhub)) + dxdt6 * cos(x6) * (sin(x10) * cos(ttilt) * Lhub + cos(x10) * (-LNaz + sin(ttilt) * Lhub)) + sin(x6) * (dxdt10 * cos(x10) * cos(ttilt) * Lhub - dxdt10 * sin(x10) * (-LNaz + sin(ttilt) * Lhub))) - dxdt5 * sin(x5) * (-Lt2 + sin(x6) * sin(x2) * La14 - sin(x6) * cos(x2) * (-cos(x10) * cos(ttilt) * Lhub + sin(x10) * (-LNaz + sin(ttilt) * Lhub)) + cos(x6) * (sin(x10) * cos(ttilt) * Lhub + cos(x10) * (-LNaz + sin(ttilt) * Lhub))) + cos(x5) * (dxdt6 * cos(x6) * sin(x2) * La14 + sin(x6) * dxdt2 * cos(x2) * La14 - dxdt6 * cos(x6) * cos(x2) * (-cos(x10) * cos(ttilt) * Lhub + sin(x10) * (-LNaz + sin(ttilt) * Lhub)) + sin(x6) * dxdt2 * sin(x2) * (-cos(x10) * cos(ttilt) * Lhub + sin(x10) * (-LNaz + sin(ttilt) * Lhub)) - sin(x6) * cos(x2) * (dxdt10 * sin(x10) * cos(ttilt) * Lhub + dxdt10 * cos(x10) * (-LNaz + sin(ttilt) * Lhub)) - dxdt6 * sin(x6) * (sin(x10) * cos(ttilt) * Lhub + cos(x10) * (-LNaz + sin(ttilt) * Lhub)) + cos(x6) * (dxdt10 * cos(x10) * cos(ttilt) * Lhub - dxdt10 * sin(x10) * (-LNaz + sin(ttilt) * Lhub)))];

% VHub02 = norm(VHub02_xyz);
% VHub04 = norm(VHub04_xyz);
% VHub12 = norm(VHub12_xyz);
% VHub14 = norm(VHub14_xyz);

VHub02 = VHub02_xyz(2);
VHub04 = VHub04_xyz(2);
VHub12 = VHub12_xyz(2);
VHub14 = VHub14_xyz(2);;
end

== matlab function NREL_20MW_4TT/4TT Tower Model/Calculate Loads ==
  data: xdotdot output; xdot output; Y input; U input; NDOF parameter; Ks parameter; Ms parameter; A_4TT parameter; B_4TT parameter; x output; F output
function [xdotdot,xdot,x,F]  = postprocess(Y,U,NDOF,Ks,Ms,A_4TT,B_4TT)

A = A_4TT;
B = B_4TT;

x=Y(1:NDOF,1);
xdot=Y(NDOF+1:NDOF*2,1);

xdotdot_temp=A*Y+B*U;
xdotdot=xdotdot_temp(NDOF+1:end,1);
% 
F=Ms*xdotdot+Ks*x;

end
